simulink model "TosAeb"  (R2021a) (continued)
== system TosAeb/FcwAebJa_Step_sys/ALG_AEBSystem/ALG_AEBOutput/AEBS_IPHUTInfoCalc ==
  note: 策略备份
  inputSignals : Inport
  latPedCalcInfo : Inport  Port=2  OutDataTypeStr=Bus: BusLatPedObjCalcInfo
  latCycCalcInfo : Inport  Port=3  OutDataTypeStr=Bus: BusLatCycObjCalcInfo
  FCWSnvtyALGInfo : Inport  Port=4
  AEBS_IPHUTInfoMap : Subsystem
  AEB_ObjStyMap : Subsystem  Commented=on
  AEB_ObjStyMap1 : Subsystem
  AEB_TrigMap : Subsystem  Commented=on
  AEB_TrigMap1 : Subsystem
  AR_HUD_InfoMap : Subsystem
  Bus Selector : BusSelector  OutputSignals=vehStateCalcInfo.VSC_AEBFuncSwtSts.AEB_VehResp,vehStateCalcInfo.VSC_AEBFuncSwtSts.AEB_PedResp,vehStateCalcInfo.VSC_AEBFuncSwtSts.FCWSnvty,vehStateCalcInfo.VSC_AEBFuncSwtSts.AEB_JAResp
  Bus Selector1 : BusSelector  OutputSignals=AEBSFuncOut.AEBTrigger,AEBModStsFuncImpArb.brkFuncImpArb.AEBpartFuncImpArb,AEBModStsFuncImpArb.brkFuncImpArb.AEBfullFuncImpArb,AEBModStsFuncImpArb.brkFuncImpArb.EBAFuncImpArb,AEBModStsFuncImpArb.brkFuncImpArb.cycAEBpartFuncImpArb,AEBModStsFuncImpArb.brkFuncImpArb.cycAEBfullFuncImpArb,AEBModStsFuncImpArb.brkFuncImpArb.pedAEBfullFuncImpArb,AEBModStsFuncImpArb.brkFuncImpArb.JA_AEBfullFuncImpArb,AEBModStsFuncImpArb.brkFuncImpArb.JA_pedAEBFuncImpArb,AEBModStsFuncImpArb.brkFuncImpArb.FCTBFuncImpArb
  Bus Selector2 : BusSelector  OutputSignals=AEBSFuncOut.AEBTrigger,longObjCalcInfo.LongObjRawInfo.ObjectType,AEBModStsFuncImpArb.brkFuncImpArb.EBAFuncImpArb,AEBModStsFuncImpArb.brkFuncImpArb.AEBfullFuncImpArb,AEBModStsFuncImpArb.brkFuncImpArb.AEBpartFuncImpArb,AEBModStsFuncImpArb.brkFuncImpArb.pedAEBfullFuncImpArb,AEBModStsFuncImpArb.brkFuncImpArb.cycAEBfullFuncImpArb,AEBModStsFuncImpArb.brkFuncImpArb.cycAEBpartFuncImpArb,AEBModStsFuncImpArb.brkFuncImpArb.JA_pedAEBFuncImpArb,AEBModStsFuncImpArb.brkFuncImpArb.JA_AEBfullFuncImpArb
  Bus Selector3 : BusSelector  OutputSignals=AEBSFuncOut.preWarningTrigger,AEBSFuncOut.latentWarningTrigger,AEBSFuncOut.AEBTrigger
  Bus Selector5 : BusSelector  OutputSignals=vehStateCalcInfo.VSC_AEBFuncSwtSts.AEB_VehResp,vehStateCalcInfo.VSC_AEBFuncSwtSts.AEB_PedResp,vehStateCalcInfo.VSC_AEBFuncSwtSts.AEB_JAResp,DiaManageInfo.FCW_AEB_ErrorState,DiaManageInfo.AEB_PEDP_ErrorState,DiaManageInfo.AEB_JA_ErrorState,VehCanInfo.EspFuncOffSts,VehCanInfo.TrailerSts
  Bus Selector6 : BusSelector  OutputSignals=longObjCalcInfo.LongCollisionAssess.TTC,vehConfigInfo.VehicleConfigAEBPed,vehConfigInfo.VehicleConfigAEBCyc,AEBModStsFuncImpArb.warningFuncImpArb.pedPreWarningFuncImpArb,AEBModStsFuncImpArb.brkReadyFuncImpArb.pedPrefillFuncImpArb,AEBModStsFuncImpArb.brkReadyFuncImpArb.pedABAFuncImpArb,AEBModStsFuncImpArb.brkFuncImpArb.pedAEBfullFuncImpArb,AEBModStsFuncImpArb.brkReadyFuncImpArb.cycPrefillFuncImpArb,AEBModStsFuncImpArb.brkFuncImpArb.cycAEBpartFuncImpArb,AEBModStsFuncImpArb.brkFuncImpArb.cycAEBfullFuncImpArb
  FCW_AEB_FctStsCalc : Subsystem
  FCW_WarnMap : Subsystem
  Terminator : Terminator
  AEB_IPHUT_INFO : Outport  OutDataTypeStr=Bus: AEB_IPHUT_INFO_BUS
  wires:
  FCW_AEB_FctStsCalc/3(AEB_JAFuncSts) -> AEBS_IPHUTInfoMap/7(FCW_AEB_JAFctSts)
  FCW_AEB_FctStsCalc/2(FCW_AEB_PedFctSts) -> AEBS_IPHUTInfoMap/6(FCW_AEB_PedFctSts)
  FCW_AEB_FctStsCalc/1(FCW_AEB_VehFctSts) -> AEBS_IPHUTInfoMap/5(FCW_AEB_VehFctSts)
  Bus Selector5/6 -> FCW_AEB_FctStsCalc/6(AEB_JA_ErrorState)  "<AEB_JA_ErrorState>"
  Bus Selector5/1 -> FCW_AEB_FctStsCalc/1(AEB_VehResp)  "<AEB_VehResp>"
  Bus Selector5/4 -> FCW_AEB_FctStsCalc/4(FCW_AEB_ErrorState)  "<FCW_AEB_ErrorState>"
  Bus Selector5/2 -> FCW_AEB_FctStsCalc/2(AEB_PedResp)  "<AEB_PedResp>"
  inputSignals/1 -> Bus Selector/1, Bus Selector2/1, Bus Selector1/1, Bus Selector3/1, Bus Selector6/1, Bus Selector5/1
  Bus Selector5/5 -> FCW_AEB_FctStsCalc/5(AEB_PEDP_ErrorState)  "<AEB_PEDP_ErrorState>"
  Bus Selector5/3 -> FCW_AEB_FctStsCalc/3(AEB_JAResp)  "<AEB_JAResp>"
  Bus Selector5/8 -> FCW_AEB_FctStsCalc/8(TrailerSts)  "<TrailerSts>"
  Bus Selector5/7 -> FCW_AEB_FctStsCalc/7(EspFuncOffSts)  "<EspFuncOffSts>"
  Bus Selector2/2 -> AEB_TrigMap1/2(AEBLonObjectType)  "<ObjectType>"
  Bus Selector6/3 -> AR_HUD_InfoMap/3(VehicleConfigAEBCyc)  "<VehicleConfigAEBCyc>"
  Bus Selector6/2 -> AR_HUD_InfoMap/2(VehicleConfigAEBPed)  "<VehicleConfigAEBPed>"
  Bus Selector1/10 -> AEB_ObjStyMap1/10(FCTBFuncImpArb)  "<FCTBFuncImpArb>"
  Bus Selector1/9 -> AEB_ObjStyMap1/9(JA_pedAEBfullFuncImpArb)  "<JA_pedAEBFuncImpArb>"
  Bus Selector1/8 -> AEB_ObjStyMap1/8(JA_AEBfullFuncImpArb)  "<JA_AEBfullFuncImpArb>"
  Bus Selector1/7 -> AEB_ObjStyMap1/7(pedAEBfullFuncImpArb)  "<pedAEBfullFuncImpArb>"
  Bus Selector1/6 -> AEB_ObjStyMap1/6(cycAEBfullFuncImpArb)  "<cycAEBfullFuncImpArb>"
  Bus Selector1/5 -> AEB_ObjStyMap1/5(cycAEBpartFuncImpArb)  "<cycAEBpartFuncImpArb>"
  Bus Selector1/4 -> AEB_ObjStyMap1/4(EBAFuncImpArb)  "<EBAFuncImpArb>"
  Bus Selector1/3 -> AEB_ObjStyMap1/3(AEBfullFuncImpArb)  "<AEBfullFuncImpArb>"
  Bus Selector1/2 -> AEB_ObjStyMap1/2(AEBpartFuncImpArb)  "<AEBpartFuncImpArb>"
  Bus Selector1/1 -> AEB_ObjStyMap1/1(AEBTrigger)  "<AEBTrigger>"
  Bus Selector6/10 -> AR_HUD_InfoMap/10(cycAEBfullFuncImpArb)  "<cycAEBfullFuncImpArb>"
  Bus Selector6/9 -> AR_HUD_InfoMap/9(cycAEBpartFuncImpArb)  "<cycAEBpartFuncImpArb>"
  Bus Selector6/8 -> AR_HUD_InfoMap/8(cycPrefillFuncImpArb)  "<cycPrefillFuncImpArb>"
  Bus Selector6/7 -> AR_HUD_InfoMap/7(pedAEBfullFuncImpArb)  "<pedAEBfullFuncImpArb>"
  Bus Selector6/6 -> AR_HUD_InfoMap/6(pedABAFuncImpArb)  "<pedABAFuncImpArb>"
  Bus Selector6/5 -> AR_HUD_InfoMap/5(pedPrefillFuncImpArb)  "<pedPrefillFuncImpArb>"
  Bus Selector6/4 -> AR_HUD_InfoMap/4(pedPreWarningFuncImpArb)  "<pedPreWarningFuncImpArb>"
  Bus Selector6/1 -> AR_HUD_InfoMap/1(FCWAEB_longTTC)  "<TTC>"
  AR_HUD_InfoMap/1(AR_HUD_Info) -> AEBS_IPHUTInfoMap/14(AR_HUD_Info)
  latCycCalcInfo/1 -> AR_HUD_InfoMap/12(latCycCalcInfo)  "latCycCalcInfo"
  latPedCalcInfo/1 -> AR_HUD_InfoMap/11(latPedCalcInfo)  "latPedCalcInfo"
  Bus Selector3/3 -> FCW_WarnMap/3(AEBTrigger)  "<AEBTrigger>"
  Bus Selector/1 -> AEBS_IPHUTInfoMap/1(AEB_VehResp)  "<AEB_VehResp>"
  Bus Selector/2 -> AEBS_IPHUTInfoMap/2(AEB_PedResp)  "<AEB_PedResp>"
  Bus Selector/4 -> AEBS_IPHUTInfoMap/4(AEB_JAResp)  "<AEB_JAResp>"
  AEB_TrigMap1/3(AEB_JABrkTrig) -> AEBS_IPHUTInfoMap/10(AEB_JABrkTrig)  "AEB_JABrkTrig"
  AEB_TrigMap1/2(AEB_PedTrig) -> AEBS_IPHUTInfoMap/9(AEB_PedTrig)  "AEB_PedTrig"
  AEB_TrigMap1/1(AEB_VehTrig) -> AEBS_IPHUTInfoMap/8(AEB_VehTrig)  "AEB_VehTrig"
  AEB_ObjStyMap1/1(AEB_ObjSty) -> AEBS_IPHUTInfoMap/11(AEB_ObjSty)  "AEB_ObjSty"
  FCW_WarnMap/1(FCW_Warn) -> AEBS_IPHUTInfoMap/12(FCW_Warn)  "FCW_Warn"
  AEBS_IPHUTInfoMap/1(AEB_IPHUT_INFO) -> AEB_IPHUT_INFO/1
  Bus Selector2/10 -> AEB_TrigMap1/10(JA_AEBfullFuncImpArb)  "<JA_AEBfullFuncImpArb>"
  Bus Selector2/9 -> AEB_TrigMap1/9(JA_pedAEBfullFuncImpArb)  "<JA_pedAEBFuncImpArb>"
  Bus Selector2/8 -> AEB_TrigMap1/8(cycAEBpartFuncImpArb)  "<cycAEBpartFuncImpArb>"
  Bus Selector2/7 -> AEB_TrigMap1/7(cycAEBfullFuncImpArb)  "<cycAEBfullFuncImpArb>"
  Bus Selector2/1 -> AEB_TrigMap1/1(AEBTrigger)  "<AEBTrigger>"
  Bus Selector2/6 -> AEB_TrigMap1/6(pedAEBfullFuncImpArb)  "<pedAEBfullFuncImpArb>"
  Bus Selector2/3 -> AEB_TrigMap1/3(EBAfullFuncImpArb)  "<EBAFuncImpArb>"
  Bus Selector2/4 -> AEB_TrigMap1/4(AEBfullFuncImpArb)  "<AEBfullFuncImpArb>"
  Bus Selector2/5 -> AEB_TrigMap1/5(AEBPartFuncImpArb)  "<AEBpartFuncImpArb>"
  Bus Selector3/1 -> FCW_WarnMap/1(preWarningTrigger)  "<preWarningTrigger>"
  Bus Selector3/2 -> FCW_WarnMap/2(latentWarningTrigger)  "<latentWarningTrigger>"
  FCW_WarnMap/2(Latten_Warn) -> AEBS_IPHUTInfoMap/13(Latten_Warn)  "Latten_Warn"
  FCWSnvtyALGInfo/1 -> AEBS_IPHUTInfoMap/3(FCWSnvty)
  Bus Selector/3 -> Terminator/1  "<FCWSnvty>"

== system TosAeb/FcwAebJa_Step_sys/ALG_AEBSystem/ALG_AEBOutput/AEB_EDR_JAPED_INFOMap ==
  VehConfigAEBJA : Inport
  JaPedTgtCalcInfo : Inport  Port=2
  Compare To Constant : simulink/Logic and Bit Operations/Compare To Constant  relop===  const=FALSE  OutDataTypeStr=boolean
  If : If  IfExpression=u1
  If Action Subsystem : Subsystem  TreatAsAtomicUnit=on
  Merge : Merge
  Subsystem : Subsystem
  AEB_EDR_JAPED_INFO : Outport  OutDataTypeStr=Bus: AEB_EDR_JAPED_INFO_BUS
  wires:
  If/2 -> Subsystem/Action
  If/1 -> If Action Subsystem/Action
  Merge/1 -> AEB_EDR_JAPED_INFO/1
  Subsystem/1(AEB_EDR_JAPED_INFO) -> Merge/2
  If Action Subsystem/1(AEB_EDR_JAPED_INFO) -> Merge/1
  VehConfigAEBJA/1 -> Compare To Constant/1
  Compare To Constant/1 -> If/1
  JaPedTgtCalcInfo/1 -> Subsystem/1(JaPedTgtCalcInfo)

== system TosAeb/FcwAebJa_Step_sys/ALG_AEBSystem/ALG_AEBOutput/AEB_EDR_JAVEH_INFOMap ==
  VehConfigAEBJA : Inport
  JaVehTgtCalcInfo : Inport  Port=2
  Compare To Constant : simulink/Logic and Bit Operations/Compare To Constant  relop===  const=FALSE  OutDataTypeStr=boolean
  If : If  IfExpression=u1
  If Action Subsystem : Subsystem  TreatAsAtomicUnit=on
  Merge : Merge
  Subsystem : Subsystem
  AEB_EDR_JAVEH_INFO : Outport  OutDataTypeStr=Bus: AEB_EDR_JAVEH_INFO_BUS
  wires:
  Merge/1 -> AEB_EDR_JAVEH_INFO/1
  Subsystem/1(AEB_EDR_JAVEH_INFO) -> Merge/2
  If Action Subsystem/1(AEB_EDR_JAVEH_INFO) -> Merge/1
  JaVehTgtCalcInfo/1 -> Subsystem/1(JaVehTgtCalcInfo)
  If/2 -> Subsystem/Action
  If/1 -> If Action Subsystem/Action
  Compare To Constant/1 -> If/1
  VehConfigAEBJA/1 -> Compare To Constant/1

== system TosAeb/FcwAebJa_Step_sys/ALG_AEBSystem/ALG_AEBOutput/AEB_EDR_LATCYC_INFOMap ==
  VehicleConfigAEBCyc : Inport
  latCycTgtCalcInfo : Inport  Port=2
  Compare To Constant : simulink/Logic and Bit Operations/Compare To Constant  relop===  const=FALSE  OutDataTypeStr=boolean
  If : If  IfExpression=u1
  If Action Subsystem : Subsystem  TreatAsAtomicUnit=on
  Merge : Merge
  Subsystem : Subsystem
  AEB_EDR_LATCYC_INFO : Outport  OutDataTypeStr=Bus: AEB_EDR_LATCYC_INFO_BUS
  wires:
  VehicleConfigAEBCyc/1 -> Compare To Constant/1
  Merge/1 -> AEB_EDR_LATCYC_INFO/1
  Subsystem/1(AEB_EDR_LATCYC_INFO) -> Merge/2
  If Action Subsystem/1(AEB_EDR_LATCYC_INFO) -> Merge/1
  If/2 -> Subsystem/Action
  If/1 -> If Action Subsystem/Action
  Compare To Constant/1 -> If/1
  latCycTgtCalcInfo/1 -> Subsystem/1(latCycTgtCalcInfo)

== system TosAeb/FcwAebJa_Step_sys/ALG_AEBSystem/ALG_AEBOutput/AEB_EDR_LATPED_INFOMap ==
  VehicleConfigAEBPed : Inport
  latPedTgtCalcInfo : Inport  Port=2
  Compare To Constant : simulink/Logic and Bit Operations/Compare To Constant  relop===  const=FALSE  OutDataTypeStr=boolean
  If : If  IfExpression=u1
  If Action Subsystem : Subsystem  TreatAsAtomicUnit=on
  Merge : Merge
  Subsystem : Subsystem
  AEB_EDR_LATPED_INFO : Outport  OutDataTypeStr=Bus: AEB_EDR_LATPED_INFO_BUS
  wires:
  If/2 -> Subsystem/Action
  Merge/1 -> AEB_EDR_LATPED_INFO/1
  Subsystem/1(AEB_EDR_LATPED_INFO) -> Merge/2
  If Action Subsystem/1(AEB_EDR_LATPED_INFO) -> Merge/1
  latPedTgtCalcInfo/1 -> Subsystem/1(latPedTgtCalcInfo)
  If/1 -> If Action Subsystem/Action
  VehicleConfigAEBPed/1 -> Compare To Constant/1
  Compare To Constant/1 -> If/1

== system TosAeb/FcwAebJa_Step_sys/ALG_AEBSystem/ALG_AEBOutput/AEB_EDR_LONOBJ_INFOMap ==
  VehicleConfigFCW : Inport
  VehicleConfigAEB : Inport  Port=2
  longObjCalcInfo : Inport  Port=3
  Compare To Constant : simulink/Logic and Bit Operations/Compare To Constant  relop===  const=FALSE  OutDataTypeStr=boolean
  If : If  IfExpression=u1
  If Action Subsystem : Subsystem  TreatAsAtomicUnit=on
  Logical Operator : Logic  Operator=OR  AllPortsSameDT=off  OutDataTypeStr=boolean
  Merge : Merge
  Subsystem : Subsystem
  AEB_EDR_LONOBJ_INFO : Outport  OutDataTypeStr=Bus: AEB_EDR_LONOBJ_INFO_BUS
  wires:
  VehicleConfigAEB/1 -> Logical Operator/2
  VehicleConfigFCW/1 -> Logical Operator/1
  Merge/1 -> AEB_EDR_LONOBJ_INFO/1
  Subsystem/1(AEB_EDR_LONOBJ_INFO) -> Merge/2
  If Action Subsystem/1(AEB_EDR_LONOBJ_INFO) -> Merge/1
  If/2 -> Subsystem/Action
  If/1 -> If Action Subsystem/Action
  longObjCalcInfo/1 -> Subsystem/1(longObjCalcInfo)
  Logical Operator/1 -> Compare To Constant/1
  Compare To Constant/1 -> If/1

== system TosAeb/FcwAebJa_Step_sys/ALG_AEBSystem/ALG_AEBOutput/AEB_FUNC_STS_INFOMap ==
  AEBModStsFuncImpArb : Inport  OutDataTypeStr=Bus: BusAEBModStsFuncImpArb
  Bus Creator : BusCreator  Inputs=26  OutDataTypeStr=Bus: AEB_FUNC_STS_INFO_BUS  NonVirtualBus=on
      settings: InheritFromInputs=off
  Bus Selector : BusSelector  OutputSignals=warningFuncImpArb.latentWarningFuncImpArb,warningFuncImpArb.preWarningFuncImpArb,warningFuncImpArb.acuteWarningFuncImpArb,warningFuncImpArb.pedPreWarningFuncImpArb,brkReadyFuncImpArb.prefillFuncImpArb,brkReadyFuncImpArb.ABAFuncImpArb,brkReadyFuncImpArb.pedPrefillFuncImpArb,brkReadyFuncImpArb.pedABAFuncImpArb,brkReadyFuncImpArb.cycPrefillFuncImpArb,brkReadyFuncImpArb.JA_vehPrefillFuncImpArb,brkReadyFuncImpArb.JA_PTW_PrefillFuncImpArb,brkReadyFuncImpArb.JA_cycPrefillFuncImpArb,brkReadyFuncImpArb.JA_pedPrefillFuncImpArb,brkReadyFuncImpArb.FCTB_PrefillFuncImpArb,brkReadyFuncImpArb.FCTB_ABAFuncImpArb,brkFuncImpArb.AEBpartFuncImpArb,brkFuncImpArb.cycAEBpartFuncImpArb,brkFuncImpArb.AEBfullFuncImpArb,brkFuncImpArb.JA_AEBfullFuncImpArb,brkFuncImpArb.cycAEBfullFuncImpArb,brkFuncImpArb.pedAEBfullFuncImpArb,brkFuncImpArb.EBAFuncImpArb,brkFuncImpArb.FCTBFuncImpArb,brkFuncImpArb.JA_PTW_AEBFuncImpArb,brkFuncImpArb.JA_cycAEBFuncImpArb,brkFuncImpArb.JA_pedAEBFuncImpArb
  Data Type Conversion{,1..25} (26 alike) : DataTypeConversion  OutDataTypeStr=uint8
  AEB_FUNC_STS_INFO : Outport  OutDataTypeStr=Bus: AEB_FUNC_STS_INFO_BUS
  wires:
  Data Type Conversion25/1 -> Bus Creator/26  "JA_PedAEBFuncSts"
  Data Type Conversion24/1 -> Bus Creator/25  "JA_CycAEBFuncSts"
  Data Type Conversion22/1 -> Bus Creator/17  "CycAEBpartFuncSts"
  Data Type Conversion21/1 -> Bus Creator/24  "JA_PTW_AEBFuncSts"
  Data Type Conversion23/1 -> Bus Creator/18  "AEBfullFuncSts"
  Data Type Conversion17/1 -> Bus Creator/20  "CycAEBfullFuncSts"
  Data Type Conversion19/1 -> Bus Creator/22  "EBAFuncSts"
  Data Type Conversion16/1 -> Bus Creator/19  "JA_AEBfullFuncSts"
  Data Type Conversion18/1 -> Bus Creator/21  "PedAEBfullFuncSts"
  Data Type Conversion20/1 -> Bus Creator/23  "FCTBFuncSts"
  Data Type Conversion14/1 -> Bus Creator/15  "FCTB_ABAFuncSts"
  Data Type Conversion9/1 -> Bus Creator/10  "JA_VehPrefillFuncSts"
  Data Type Conversion11/1 -> Bus Creator/12  "JA_CycPrefillFuncSts"
  Data Type Conversion13/1 -> Bus Creator/14  "FCTB_PrefillFuncSts"
  Data Type Conversion12/1 -> Bus Creator/13  "JA_PedPrefillFuncSts"
  Data Type Conversion10/1 -> Bus Creator/11  "JA_PTW_PrefillFuncSts"
  Data Type Conversion8/1 -> Bus Creator/9  "CycPrefillFuncSts"
  Data Type Conversion15/1 -> Bus Creator/16  "AEBpartFuncSts"
  Data Type Conversion7/1 -> Bus Creator/8  "PedABAFuncSts"
  Data Type Conversion5/1 -> Bus Creator/6  "ABAFuncFuncSts"
  Data Type Conversion6/1 -> Bus Creator/7  "PedPrefillFuncSts"
  Data Type Conversion4/1 -> Bus Creator/5  "PrefillFuncSts"
  Data Type Conversion3/1 -> Bus Creator/4  "PedPreWarningFuncSts"
  Data Type Conversion2/1 -> Bus Creator/3  "AcuteWarningFuncSts"
  Data Type Conversion1/1 -> Bus Creator/2  "PreWarningFuncSts"
  Data Type Conversion/1 -> Bus Creator/1  "LatentWarningFuncSts"
  Bus Selector/26 -> Data Type Conversion25/1  "<JA_pedAEBFuncImpArb>"
  Bus Selector/25 -> Data Type Conversion24/1  "<JA_cycAEBFuncImpArb>"
  Bus Selector/24 -> Data Type Conversion21/1  "<JA_PTW_AEBFuncImpArb>"
  Bus Selector/23 -> Data Type Conversion20/1  "<FCTBFuncImpArb>"
  Bus Selector/22 -> Data Type Conversion19/1  "<EBAFuncImpArb>"
  Bus Selector/21 -> Data Type Conversion18/1  "<pedAEBfullFuncImpArb>"
  Bus Selector/20 -> Data Type Conversion17/1  "<cycAEBfullFuncImpArb>"
  Bus Selector/19 -> Data Type Conversion16/1  "<JA_AEBfullFuncImpArb>"
  Bus Selector/18 -> Data Type Conversion23/1  "<AEBfullFuncImpArb>"
  Bus Selector/17 -> Data Type Conversion22/1  "<cycAEBpartFuncImpArb>"
  Bus Selector/16 -> Data Type Conversion15/1  "<AEBpartFuncImpArb>"
  Bus Selector/15 -> Data Type Conversion14/1  "<FCTB_ABAFuncImpArb>"
  Bus Selector/14 -> Data Type Conversion13/1  "<FCTB_PrefillFuncImpArb>"
  Bus Selector/13 -> Data Type Conversion12/1  "<JA_pedPrefillFuncImpArb>"
  Bus Selector/12 -> Data Type Conversion11/1  "<JA_cycPrefillFuncImpArb>"
  Bus Selector/11 -> Data Type Conversion10/1  "<JA_PTW_PrefillFuncImpArb>"
  Bus Selector/10 -> Data Type Conversion9/1  "<JA_vehPrefillFuncImpArb>"
  Bus Selector/9 -> Data Type Conversion8/1  "<cycPrefillFuncImpArb>"
  Bus Selector/8 -> Data Type Conversion7/1  "<pedABAFuncImpArb>"
  Bus Selector/7 -> Data Type Conversion6/1  "<pedPrefillFuncImpArb>"
  Bus Selector/6 -> Data Type Conversion5/1  "<ABAFuncImpArb>"
  Bus Selector/5 -> Data Type Conversion4/1  "<prefillFuncImpArb>"
  Bus Selector/4 -> Data Type Conversion3/1  "<pedPreWarningFuncImpArb>"
  Bus Selector/3 -> Data Type Conversion2/1  "<acuteWarningFuncImpArb>"
  Bus Selector/2 -> Data Type Conversion1/1  "<preWarningFuncImpArb>"
  Bus Selector/1 -> Data Type Conversion/1  "<latentWarningFuncImpArb>"
  Bus Creator/1 -> AEB_FUNC_STS_INFO/1
  AEBModStsFuncImpArb/1 -> Bus Selector/1

== system TosAeb/AEB_Objsel/TA_InfoRebuild/TAObjInfoCalc/For Each Subsystem/ForEachInput ==
  Index : Inport
  Cam_laneLeftPortion : Inport  Port=2
  Cam_laneAssociateConf : Inport  Port=3
  Cam_laneRightPortion : Inport  Port=4
  Cam_laneLeftConf : Inport  Port=5
  Cam_laneRightConf : Inport  Port=6
  Cam_objIntention : Inport  Port=7
  Cam_objIntentionProb : Inport  Port=8
  Cam_laneAssign : Inport  Port=9
  Cam_ObsPosX_s : Inport  Port=10
  Cam_ObsPosY_s : Inport  Port=11
  Cam_ObsAccY_s : Inport  Port=12
  Cam_ObsAccX_s : Inport  Port=13
  Cam_ObsVelY_s : Inport  Port=14
  Cam_ObsVelX_s : Inport  Port=15
  Cam_ObsID_u8 : Inport  Port=16
  Cam_ObsValid_u8 : Inport  Port=17
  Bus Creator2 : BusCreator  Inputs=16
  Index Vector{,1..15} (16 alike) : MultiPortSwitch  DataPortOrder=Zero-based contiguous  Inputs=1  zeroidx=on
  Obj_Infomation : Outport
  wires:
  Index Vector2/1 -> Bus Creator2/3  "laneRightPortion"
  [ID] -> Index Vector5/1
  Cam_laneLeftPortion/1 -> Index Vector/2
  Index/1 -> Index Vector/1, [ID]
  [ID] -> Index Vector1/1
  [ID] -> Index Vector10/1
  [ID] -> Index Vector2/1
  [ID] -> Index Vector3/1
  [ID] -> Index Vector6/1
  [ID] -> Index Vector4/1
  [ID] -> Index Vector7/1
  [ID] -> Index Vector8/1
  [ID] -> Index Vector9/1
  Index Vector10/1 -> Bus Creator2/11  "AccY_s"
  [ID] -> Index Vector11/1
  [ID] -> Index Vector12/1
  [ID] -> Index Vector13/1
  Index Vector/1 -> Bus Creator2/1  "laneLeftPortion"
  Index Vector9/1 -> Bus Creator2/10  "PosY_s"
  Index Vector1/1 -> Bus Creator2/2  "laneAssociateConf"
  Index Vector3/1 -> Bus Creator2/4  "laneLeftConf"
  Index Vector4/1 -> Bus Creator2/5  "laneRightConf"
  Index Vector5/1 -> Bus Creator2/6  "objIntention"
  Index Vector6/1 -> Bus Creator2/7  "objIntentionProb"
  Index Vector7/1 -> Bus Creator2/8  "laneAssign"
  Index Vector8/1 -> Bus Creator2/9  "PosX_s"
  Index Vector11/1 -> Bus Creator2/12  "AccX_s"
  Index Vector12/1 -> Bus Creator2/13  "VelY_s"
  Index Vector13/1 -> Bus Creator2/14  "VelX_s"
  Bus Creator2/1 -> Obj_Infomation/1
  Cam_laneAssociateConf/1 -> Index Vector1/2
  Cam_laneRightPortion/1 -> Index Vector2/2
  Cam_laneLeftConf/1 -> Index Vector3/2
  Cam_laneRightConf/1 -> Index Vector4/2
  Cam_objIntention/1 -> Index Vector5/2
  Cam_objIntentionProb/1 -> Index Vector6/2
  Cam_laneAssign/1 -> Index Vector7/2
  Cam_ObsPosX_s/1 -> Index Vector8/2
  Cam_ObsPosY_s/1 -> Index Vector9/2
  Cam_ObsAccY_s/1 -> Index Vector10/2
  Cam_ObsAccX_s/1 -> Index Vector11/2
  Cam_ObsVelY_s/1 -> Index Vector12/2
  Cam_ObsVelX_s/1 -> Index Vector13/2
  Index Vector14/1 -> Bus Creator2/15  "ID"
  Cam_ObsID_u8/1 -> Index Vector14/2
  [ID] -> Index Vector14/1
  Index Vector15/1 -> Bus Creator2/16  "Valid"
  Cam_ObsValid_u8/1 -> Index Vector15/2
  [ID] -> Index Vector15/1

== system TosAeb/AEB_Objsel/TA_InfoRebuild/TAObjInfoCalc/For Each Subsystem/Info_rebuild ==
  Obj_Infomation : Inport
  Road_Info : Inport  Port=2
  Basic_Info : Subsystem
  Road_relative : Subsystem
  vxRel : Outport
  vyRel : Outport  Port=2
  vxRelStd : Outport  Port=3
  vyRelStd : Outport  Port=4
  axRel : Outport  Port=5
  ayRel : Outport  Port=6
  axRelStd : Outport  Port=7
  ayRelStd : Outport  Port=8
  azimuthAngle : Outport  Port=9
  azimuthAngleStd : Outport  Port=10
  objLatDist2LaneCenter : Outport  Port=11
  objLatDist2EgoCenter : Outport  Port=12
  objPosition : Outport  Port=13
  wires:
  Basic_Info/9(azimuthAngle) -> azimuthAngle/1
  Basic_Info/7(axRelStd) -> axRelStd/1
  Obj_Infomation/1 -> Road_relative/1(Obj_Infomation), Basic_Info/1(Obj_Infomation)
  Road_Info/1 -> Road_relative/2(Road_Info)
  Basic_Info/2(vyRel) -> vyRel/1
  Basic_Info/1(vxRel) -> vxRel/1
  Basic_Info/3(vxRelStd) -> vxRelStd/1
  Basic_Info/4(vyRelStd) -> vyRelStd/1
  Basic_Info/5(axRel) -> axRel/1
  Basic_Info/8(ayRelStd) -> ayRelStd/1
  Basic_Info/6(ayRel) -> ayRel/1
  Basic_Info/10(azimuthAngleStd) -> azimuthAngleStd/1
  Road_relative/1(objLatDist2LaneCenter) -> objLatDist2LaneCenter/1
  Road_relative/2(objLatDist2EgoCenter) -> objLatDist2EgoCenter/1
  Road_relative/3(objPosition) -> objPosition/1

== system TosAeb/AEB_Objsel/TA_Processor/AEB_ObjSelect/TargetInfoCheck/Data_precess ==
  TA_ObjID : Inport
  TA_ObjType : Inport  Port=2
  TA_ObjState : Inport  Port=3
  TA_ObjOrientation : Inport  Port=4
  TA_ObjfusType : Inport  Port=5
  TA_ObjLaneAssign : Inport  Port=6
  TA_ObjDx : Inport  Port=7
  TA_ObjDy : Inport  Port=8
  TA_ObjDxStd : Inport  Port=9
  TA_ObjDyStd : Inport  Port=10
  TA_ObjVx : Inport  Port=11
  TA_ObjVy : Inport  Port=12
  TA_ObjVxStd : Inport  Port=13
  TA_ObjVyStd : Inport  Port=14
  TA_ObjVxAbs : Inport  Port=15
  TA_ObjVyAbs : Inport  Port=16
  TA_ObjLength : Inport  Port=17
  TA_ObjWidth : Inport  Port=18
  TA_ObjHight : Inport  Port=19
  TA_ObjAx : Inport  Port=20
  TA_ObjAy : Inport  Port=21
  TA_ObjAxAbs : Inport  Port=22
  TA_ObjAyAbs : Inport  Port=23
  TA_ObjConfidence : Inport  Port=24
  TA_ObjCenterAngle : Inport  Port=25
  TA_ObjHeadAngle : Inport  Port=26
  TA_ObjHeadAngleStd : Inport  Port=27
  IDForeach : Inport  Port=28  Partition=on  PartitionDimension=1  PartitionWidth=1  PartitionOffset=0
  VehSelfData : Inport  Port=29
  PathEstimn : Inport  Port=30
  For Each : ForEach  DisableCoverage=on  ShowIterationIndex=on
  ObjInfo_Assignment : Subsystem
  ObjInfo_Select : Subsystem
  TA_EffectCalculate : Subsystem
  Terminator : Terminator
  Boudingbox_Calculate : Outport  ConcatenationDimension=1
  Obj_Coef : Outport  Port=2  ConcatenationDimension=1
  TTI : Outport  Port=3  ConcatenationDimension=1
  LatObj_Valid : Outport  Port=4  ConcatenationDimension=1
  Lat_ObjIntersect : Outport  Port=5  ConcatenationDimension=1
  Lat_TTR : Outport  Port=6  ConcatenationDimension=1
  Overlap_Flg : Outport  Port=7  ConcatenationDimension=1
  JA_TTRAssign : Outport  Port=8  ConcatenationDimension=1
  Utility : Outport  Port=9  ConcatenationDimension=1
  Meas_WeightCoef_1 : Outport  Port=10  ConcatenationDimension=1
  Meas_WeightCoef_2 : Outport  Port=11  ConcatenationDimension=1
  wires:
  ObjInfo_Assignment/6(Lat_TTRAssign) -> Lat_TTR/1
  TA_EffectCalculate/4(TTI) -> ObjInfo_Assignment/4(TTI)
  TA_EffectCalculate/6(Lat_ObjIntersect) -> ObjInfo_Assignment/6(Lat_ObjIntersect)
  ObjInfo_Assignment/7(Overlap_FlgAssign) -> Overlap_Flg/1
  ObjInfo_Assignment/4(LatObj_ValidAssign) -> LatObj_Valid/1
  TA_EffectCalculate/3(Obj_Coef) -> ObjInfo_Assignment/3(Obj_Coef)
  ObjInfo_Assignment/3(TTIAssign) -> TTI/1
  TA_EffectCalculate/5(LatObj_Valid) -> ObjInfo_Assignment/5(LatObj_Valid)
  ObjInfo_Select/1(TA_ObsInfo) -> TA_EffectCalculate/1(TA_ObsInfo)
  TA_EffectCalculate/2(Boudingbox_Calculate) -> ObjInfo_Assignment/2(Boudingbox_Calculate)
  ObjInfo_Assignment/2(Obj_CoefAssign) -> Obj_Coef/1
  TA_EffectCalculate/1(Overlap_Flg) -> ObjInfo_Assignment/1(Overlap_Flg)
  TA_EffectCalculate/7(Lat_TTR) -> ObjInfo_Assignment/7(Lat_TTR)
  ObjInfo_Assignment/5(Lat_ObjIntersectAssign) -> Lat_ObjIntersect/1
  VehSelfData/1 -> TA_EffectCalculate/2(VehSelfData)
  PathEstimn/1 -> TA_EffectCalculate/3(PathEstimn)
  ObjInfo_Assignment/1(Boudingbox_CalculateAssign) -> Boudingbox_Calculate/1
  TA_ObjID/1 -> ObjInfo_Select/2(TA_ObjID)
  TA_ObjType/1 -> ObjInfo_Select/3(TA_ObjType)
  TA_ObjState/1 -> ObjInfo_Select/4(TA_ObjState)
  TA_ObjOrientation/1 -> ObjInfo_Select/5(TA_ObjOrientation)
  TA_ObjfusType/1 -> ObjInfo_Select/6(TA_ObjfusType)
  TA_ObjLaneAssign/1 -> ObjInfo_Select/7(TA_ObjLaneAssign)
  TA_ObjDx/1 -> ObjInfo_Select/8(TA_ObjDx)
  TA_ObjDy/1 -> ObjInfo_Select/9(TA_ObjDy)
  TA_ObjDxStd/1 -> ObjInfo_Select/10(TA_ObjDxStd)
  TA_ObjDyStd/1 -> ObjInfo_Select/11(TA_ObjDyStd)
  TA_ObjVx/1 -> ObjInfo_Select/12(TA_ObjVx)
  TA_ObjVy/1 -> ObjInfo_Select/13(TA_ObjVy)
  TA_ObjVxStd/1 -> ObjInfo_Select/14(TA_ObjVxStd)
  TA_ObjVyStd/1 -> ObjInfo_Select/15(TA_ObjVyStd)
  TA_ObjVxAbs/1 -> ObjInfo_Select/16(TA_ObjVxAbs)
  TA_ObjVyAbs/1 -> ObjInfo_Select/17(TA_ObjVyAbs)
  TA_ObjLength/1 -> ObjInfo_Select/18(TA_ObjLength)
  TA_ObjWidth/1 -> ObjInfo_Select/19(TA_ObjWidth)
  TA_ObjHight/1 -> ObjInfo_Select/20(TA_ObjHight)
  TA_ObjAx/1 -> ObjInfo_Select/21(TA_ObjAx)
  TA_ObjAy/1 -> ObjInfo_Select/22(TA_ObjAy)
  TA_ObjAxAbs/1 -> ObjInfo_Select/23(TA_ObjAxAbs)
  TA_ObjAyAbs/1 -> ObjInfo_Select/24(TA_ObjAyAbs)
  TA_ObjConfidence/1 -> ObjInfo_Select/25(TA_ObjConfidence)
  TA_ObjCenterAngle/1 -> ObjInfo_Select/26(TA_ObjCenterAngle)
  TA_ObjHeadAngle/1 -> ObjInfo_Select/27(TA_ObjHeadAngle)
  TA_ObjHeadAngleStd/1 -> ObjInfo_Select/28(TA_ObjHeadAngleStd)
  IDForeach/1 -> Terminator/1
  TA_EffectCalculate/8(JA_TTR) -> ObjInfo_Assignment/8(JA_TTR)
  ObjInfo_Assignment/8(JA_TTRAssign) -> JA_TTRAssign/1
  TA_EffectCalculate/9(Utility) -> Utility/1
  TA_EffectCalculate/10(Meas_WeightCoef_1) -> Meas_WeightCoef_1/1
  TA_EffectCalculate/11(Meas_WeightCoef_2) -> Meas_WeightCoef_2/1
  For Each/1 -> ObjInfo_Select/1(Index)
